simulink library "LibrarySimulinkSAS"  (R2018b)
settings: Solver=ode45  StartTime=0.0  StopTime=10.0  RelTol=1e-3
undefined symbols (not defined in the file or in its folder): IdB, IdL, IdM, shmControl, ts
libraries: $bdroot x16
(part 4 of 4)

== matlab function LibrarySimulinkSAS/ISharedMemory Control/MATLAB Function1 ==
  data: u input; y output
function y = fcn(u)
y=zeros(40,1);
y(1:length(u),1)=u;

== matlab function LibrarySimulinkSAS/ISharedMemory Control/MATLAB Function2 ==
  data: u input; y output
function y = fcn(u)
y=zeros(40,1);
y(1:length(u),1)=u;

== matlab function LibrarySimulinkSAS/plantilla IApp/sizeCalc1 ==
  data: size input; y output; type input
function y = fcn(size,type)
n=length(size);
y=0;
for i=1:n
    switch type(i)
        case 'c'
            aux=size(i)*1;
        case 'C'
            aux=size(i)*1;
        case 'd'
            aux=size(i)*6;
        case 'f'
            aux=size(i)*4;
        case 'i'
            aux=size(i)*4;
        case 'I'
            aux=size(i)*4;
        otherwise %double
            aux=size(i)*6;
    end
y=y+aux;
end
end

== matlab function LibrarySimulinkSAS/UDP Data Server/MATLAB Function ==
  data: data input; size output; array output
function [size,array] = fillData(data)
n=length(data);
size=uint32(n);
array = [data;zeros(1024-n,1)];

== matlab function LibrarySimulinkSAS/Shared Memory Step synchronization/MATLAB Function2 ==
  data: cnt_0 input; y output; cnt_1 input
function y = fcn(cnt_0,cnt_1)
if(cnt_0~=cnt_1)
    y=1;
else
    y=0;
end
